simulink model "ofdm_txrx_mimo_2x"  (Simulink 6.6)
settings: Solver=VariableStepDiscrete  StartTime=0.0  StopTime=20e3  RelTol=1e-3
PreLoadFcn:
  | ofdm_rx_mimo_init
InitFcn:
  | ofdm_rx_mimo_init
StartFcn:
  | ofdm_rx_mimo_init
values from neighbouring files (symbols this model uses):
  BER_RAM_init_size = 2048    [ofdm_tx_mimo_init.m]
  CPLength = 16    [ofdm_tx_mimo_init.m]
  CRCPolynomial = hex2dec('04c11db7')    [ofdm_tx_mimo_init.m]
  freqOffset_track_filtCoef_i = hex2dec('2500')/2^32    [ofdm_rx_mimo_init.m]
  freqOffset_track_filtCoef_p = hex2dec('d0000')/2^32    [ofdm_rx_mimo_init.m]
  hi = zeros(1,length(cci))    [ofdm_rx_mimo_init.m]
  hr = zeros(1,length(ccr))    [ofdm_rx_mimo_init.m]
  max_CP_length = 16    [ofdm_tx_mimo_init.m]
  max_num_baseRateSymbols = 31    [ofdm_tx_mimo_init.m]
  max_num_subcarriers = 64    [ofdm_tx_mimo_init.m]
  max_num_trainingSymbols = 15    [ofdm_tx_mimo_init.m]
  max_numBytes = 16384    [ofdm_tx_mimo_init.m]
  max_OFDM_symbols = 2047    [ofdm_tx_mimo_init.m]
  modConstellation_qam16 = .75*[1 3 -1 -3]./3    [ofdm_tx_mimo_init.m]
  modConstellation_qam256 = 0.9375*[3 1 5 7 11 9 13 15 -3 -1 -5 -7 -11 -9 -13 -15]./15    [ofdm_tx_mimo_init.m]
  modConstellation_qam64 = 0.875*[3 1 5 7 -3 -1 -5 -7]./7    [ofdm_tx_mimo_init.m]
  modConstellation_qpsk = [1 -1]./sqrt(2)    [ofdm_tx_mimo_init.m]
  numBaseRateSymbols = 2    [ofdm_tx_mimo_init.m]
  numSubcarriers = 64    [ofdm_tx_mimo_init.m]
  numTrainingSymbols = 2    [ofdm_tx_mimo_init.m]
  pilot1_indicies = 7 + ( (64-7) * 2^16)    (= 3735559)    [ofdm_tx_mimo_init.m]
  pilot2_indicies = 21 + ( (64-21) * 2^16)    (= 2818069)    [ofdm_tx_mimo_init.m]
  pilotValue_neg = hex2dec('8000') + (2^16 * 0)    [ofdm_tx_mimo_init.m]
  pilotValue_pos = hex2dec('7FFF') + (2^16 * 0)    [ofdm_tx_mimo_init.m]
  pkt_crossCorr_thresh = 0.7    [ofdm_rx_mimo_init.m]
  pkt_energy_thresh = 0    [ofdm_rx_mimo_init.m]
  pkt_numPayloadBytes = 1400+42-6    (= 1436)    [ofdm_tx_mimo_init.m]
  pktByteNums = numHeaderBytes +  				byteIndex_numPayloadBytes(1)*2^8 +  				byteIndex_numPayloadBytes(2)*2^16 +  				byteIndex_simpleDynModMasks * 2^24    [ofdm_rx_mimo_init.m]
  PktDet_Delay = 26 + Symbol_Timing_Offset*2^7    (= 1050)    [ofdm_rx_mimo_init.m]
  preamble = 6*[0 shortsyms_10 longsyms_2]    [ofdm_tx_mimo_init.m]
  preamble_I = real(preamble)    [ofdm_tx_mimo_init.m]
  preamble_Q = imag(preamble)    [ofdm_tx_mimo_init.m]
  RAM_init_size = max_numBytes    (= 16384)    [ofdm_tx_mimo_init.m]
  reg_Interrupt_PktBuf_Control = reg_InterruptControl +  	1 * 2^16 +  	0 * 2^24    (= 65656)    [ofdm_rx_mimo_init.m]
  rx_controlBits = 1 * 2  		 + 1 * 4  		 + 0 * 8  		 + 1 * 16 * rx_SISO_Mode  		 + 1 * 32  		 + 1 * 64  		 + 0 * 128  		 + 1 * 256  		 + 0 * 512  		 + 1 * 1024  		 + 0 * 2048  		 + 1 * 4096  		 + 1 * 8192  		 + 0 * 16384  		 + 1 * 65536  		 + 1 * 131072  		 + 0 * 262144  		 + 1 * 524288    [ofdm_rx_mimo_init.m]
  Rx_PhaseNoiseTrack_K = 0.6094    [ofdm_rx_mimo_init.m]
  rx_postEq_scaling = round(rxScaling*2^11) * (1 + 2^16)    [ofdm_rx_mimo_init.m]
  RxDataScrambling_Seq = [40 198 78 63 82 173 102 245 48 111 172 115 147 230 216 93 72 65 62 2 205 242 122 90 128 83 105 97 73 10 5 252]    [ofdm_tx_mimo_init.m]
  simOnly_numSamples = length(preamble)+( (numSubcarriers+CPLength)*(numTrainingSymbols + numBaseRateSymbols + numFullRateSymbols/2) )    [ofdm_tx_mimo_init.m]
  train = [train train]    [ofdm_tx_mimo_init.m]
  tx_controlBits = (antB_preambleShift * 2^4) + (2^0 * tx_SISO_Mode) + 4*0 + 8*1    (= 73)    [ofdm_tx_mimo_init.m]
  TxDataScrambling_Seq = [40 198 78 63 82 173 102 245 48 111 172 115 147 230 216 93 72 65 62 2 205 242 122 90 128 83 105 97 73 10 5 252]    [ofdm_tx_mimo_init.m]
  txReg_symbolCounts = (2^16 * numFullRateSymbols) + (2^8 *numBaseRateSymbols ) + numTrainingSymbols    [ofdm_tx_mimo_init.m]
  TxRx_FFTScaling = bin2dec('010111') + (bin2dec('000101') * 2^6)    [ofdm_tx_mimo_init.m]
undefined symbols (not defined in the file or in its folder): AntA, AntB, BaseRate, CRC_table_gen, gcbh, intfilt, pktTiming_count_payloadStart, pktTiming_symbol_length, xlBlockLoadCallback, xlGetSimulinkPeriod
libraries: xbsIndex_r4 x3973, dspsigops x4, commchan3 x2, commrflib2 x2, dspsrcs4 x1
(part 4 of 38)

== system ofdm_txrx_mimo_2x/Memory-mapped Registers/Tx Registers ==
  note: LSB
  note: 0x1
  note: 0x2
  note: 0x2
  note: 0x4
  note: 0x8
  note: 0xF0
  note: 0x100
  note: 0x1
  Down Sample{1,3..5} (4 alike) : xbsIndex_r4/Down Sample  sample_ratio=2  sample_phase="Last Value of Frame  (most efficient)"  en=off  latency=1  dbl_ovrd=off  block_type=dsamp
      settings: block_version=VER_STRING_GOES_HERE
  Down Sample2 : xbsIndex_r4/Down Sample  sample_ratio=4  sample_phase="Last Value of Frame  (most efficient)"  en=off  latency=1  dbl_ovrd=off  block_type=dsamp
      settings: block_version=VER_STRING_GOES_HERE
  Read Only Register : Subsystem  AncestorBlock=|  mask{regName='Tx_PktRunning'}
      | WARP_Blockset/Register Wrappers
      | (Sysgen 10.1+)/Read Only Register
      mask prompts: regName "Register Name"
      mask help:
      | This block is a wrapper for System Generator's To Register shared memory block. This register is designed for use with WARP's sysgen2opb tool.
      |
      | Note: The Regisger Name paramter below must be unique for every instance of Read-Write and Read-Only registers in your system.
      mask init: same as ofdm_txrx_mimo_2x/Memory-mapped Registers/Rx Registers/Read Only Register
      settings: AttributesFormatString=RO Reg: %<regName>\n%<ReferenceBlock>
  Read-Write Register : Subsystem  AncestorBlock=|  mask{regName='Tx_Start_Reset_Control', out_dataType=Unsigned, out_numBits=32, out_binaryPt=0, initValue=0, sampPeriod=1}
      | WARP_Blockset/Register Wrappers
      | (Sysgen 10.1+)/Read-Write Register
      mask prompts: regName "Register Name"; out_dataType "Output Type"; out_numBits "Output Num Bits"; out_binaryPt "Output Binary Point"; initValue "Initial Value"; sampPeriod "Sample Period"
      mask help:
      | This block is a wrapper for two registers from the System Generator Shared Memory blockset:
      | a) A "From Register" block, which provides a write-only register for the host processor
      | b) A "To Register" block, which provides a read-only register for the host processor
      |
      | Note: The Regisger Name paramter below must be unique for every instance of shared-memory registers in your system. System Generator will throw an error if duplicate names are found.
      mask init: same as ofdm_txrx_mimo_2x/Memory-mapped Registers/Rx Registers/Read-Write Register
      settings: AttributesFormatString=RW Reg: %<regName>
      mask StyleString: edit,popup(Unsigned|Signed  (2's comp)),edit,edit,edit,edit
  Read-Write Register1 : Subsystem  AncestorBlock=|  mask{regName='Tx_ControlBits', out_dataType=Unsigned, out_numBits=32, out_binaryPt=0, initValue=tx_controlBits, sampPeriod=1}
      | WARP_Blockset/Register Wrappers
      | (Sysgen 10.1+)/Read-Write Register
      mask prompts: regName "Register Name"; out_dataType "Output Type"; out_numBits "Output Num Bits"; out_binaryPt "Output Binary Point"; initValue "Initial Value"; sampPeriod "Sample Period"
      mask help:
      | This block is a wrapper for two registers from the System Generator Shared Memory blockset:
      | a) A "From Register" block, which provides a write-only register for the host processor
      | b) A "To Register" block, which provides a read-only register for the host processor
      |
      | Note: The Regisger Name paramter below must be unique for every instance of shared-memory registers in your system. System Generator will throw an error if duplicate names are found.
      mask init: same as ofdm_txrx_mimo_2x/Memory-mapped Registers/Rx Registers/Read-Write Register
      settings: AttributesFormatString=RW Reg: %<regName>
      mask StyleString: edit,popup(Unsigned|Signed  (2's comp)),edit,edit,edit,edit
  Read-Write Register2 : Subsystem  AncestorBlock=|  mask{regName='Tx_OFDM_SymCounts', out_dataType=Unsigned, out_numBits=32, out_binaryPt=0, initValue=txReg_symbolCounts, sampPeriod=1}
      | WARP_Blockset/Register Wrappers
      | (Sysgen 10.1+)/Read-Write Register
      mask prompts: regName "Register Name"; out_dataType "Output Type"; out_numBits "Output Num Bits"; out_binaryPt "Output Binary Point"; initValue "Initial Value"; sampPeriod "Sample Period"
      mask help:
      | This block is a wrapper for two registers from the System Generator Shared Memory blockset:
      | a) A "From Register" block, which provides a write-only register for the host processor
      | b) A "To Register" block, which provides a read-only register for the host processor
      |
      | Note: The Regisger Name paramter below must be unique for every instance of shared-memory registers in your system. System Generator will throw an error if duplicate names are found.
      mask init: same as ofdm_txrx_mimo_2x/Memory-mapped Registers/Rx Registers/Read-Write Register
      settings: AttributesFormatString=RW Reg: %<regName>
      mask StyleString: edit,popup(Unsigned|Signed  (2's comp)),edit,edit,edit,edit
  Reinterpret : xbsIndex_r4/Reinterpret  force_arith_type=on  arith_type=Unsigned  force_bin_pt=on  bin_pt=16  block_type=reinterpret
      settings: infoedit="Changes signal type without altering the binary representation.   You can changed the signal between signed and unsigned, and relocate the binary point.<P><P>Hardware notes: In hardware this block costs nothing.<P><P>Example:  Suppose the input is 6 bits wide, signed, with 2 fractional bits, and the output is forced to unsigned with 0 fractional bits.  Then an input of -2.0 (1110.00 in binary 2's complement) becomes an output of 56 (111000 in binary)."  block_version=10.1
  Slice1 : xbsIndex_r4/Slice  nbits=1  boolean_output=on  mode=Lower Bit Location + Width  bit1=0  base1=MSB of Input  bit0=0  base0=LSB of Input  dbl_ovrd=off  block_type=slice
      settings: infoedit="Extracts a given range of bits from each input sample and presents it at the output.  The output type is ordinarily unsigned with binary point at zero, but can be Boolean when the slice is one bit wide.<P><P>Hardware notes: In hardware this block costs nothing."  block_version=VER_STRING_GOES_HERE
  Slice14 : xbsIndex_r4/Slice  nbits=1  boolean_output=off  mode=Lower Bit Location + Width  bit1=0  base1=MSB of Input  bit0=0  base0=LSB of Input  dbl_ovrd=off  block_type=slice
      settings: infoedit="Extracts a given range of bits from each input sample and presents it at the output.  The output type is ordinarily unsigned with binary point at zero, but can be Boolean when the slice is one bit wide.<P><P>Hardware notes: In hardware this block costs nothing."  block_version=VER_STRING_GOES_HERE
  Slice15 : xbsIndex_r4/Slice  nbits=1  boolean_output=on  mode=Lower Bit Location + Width  bit1=0  base1=MSB of Input  bit0=1  base0=LSB of Input  dbl_ovrd=off  block_type=slice
      settings: infoedit="Extracts a given range of bits from each input sample and presents it at the output.  The output type is ordinarily unsigned with binary point at zero, but can be Boolean when the slice is one bit wide.<P><P>Hardware notes: In hardware this block costs nothing."  block_version=VER_STRING_GOES_HERE
  Slice3 : xbsIndex_r4/Slice  nbits=1  boolean_output=on  mode=Lower Bit Location + Width  bit1=0  base1=MSB of Input  bit0=2  base0=LSB of Input  dbl_ovrd=off  block_type=slice
      settings: infoedit="Extracts a given range of bits from each input sample and presents it at the output.  The output type is ordinarily unsigned with binary point at zero, but can be Boolean when the slice is one bit wide.<P><P>Hardware notes: In hardware this block costs nothing."  block_version=VER_STRING_GOES_HERE
  Slice4 : xbsIndex_r4/Slice  nbits=1  boolean_output=on  mode=Lower Bit Location + Width  bit1=0  base1=MSB of Input  bit0=3  base0=LSB of Input  dbl_ovrd=off  block_type=slice
      settings: infoedit="Extracts a given range of bits from each input sample and presents it at the output.  The output type is ordinarily unsigned with binary point at zero, but can be Boolean when the slice is one bit wide.<P><P>Hardware notes: In hardware this block costs nothing."  block_version=VER_STRING_GOES_HERE
  Slice5 : xbsIndex_r4/Slice  nbits=4  boolean_output=off  mode=Lower Bit Location + Width  bit1=0  base1=MSB of Input  bit0=4  base0=LSB of Input  dbl_ovrd=off  block_type=slice
      settings: infoedit="Extracts a given range of bits from each input sample and presents it at the output.  The output type is ordinarily unsigned with binary point at zero, but can be Boolean when the slice is one bit wide.<P><P>Hardware notes: In hardware this block costs nothing."  block_version=VER_STRING_GOES_HERE
  Slice6 : xbsIndex_r4/Slice  nbits=1  boolean_output=off  mode=Lower Bit Location + Width  bit1=0  base1=MSB of Input  bit0=8  base0=LSB of Input  dbl_ovrd=off  block_type=slice
      settings: infoedit="Extracts a given range of bits from each input sample and presents it at the output.  The output type is ordinarily unsigned with binary point at zero, but can be Boolean when the slice is one bit wide.<P><P>Hardware notes: In hardware this block costs nothing."  block_version=VER_STRING_GOES_HERE
  Write-Only Register : Subsystem  AncestorBlock=|  mask{regName='Tx_PreambleMasking', out_dataType=Unsigned, out_numBits=32, out_binaryPt=0, initValue=0, sampPeriod=1}
      | WARP_Blockset/Register Wrappers
      | (Sysgen 10.1+)/Write-Only Register
      mask prompts: regName "Register Name"; out_dataType "Output Type"; out_numBits "Output Num Bits"; out_binaryPt "Output Binary Point"; initValue "Initial Value"; sampPeriod "Sample Period"
      mask help:
      | This block is a wrapper for System Generator's "From Register" shared memory block.
      |
      | Note: The Regisger Name paramter below must be unique for every instance of shared-memory registers in your system. System Generator will throw an error if duplicate names are found.
      mask init: same as ofdm_txrx_mimo_2x/Memory-mapped Registers/Rx Registers/Write-Only Register
      settings: AttributesFormatString=RW Reg: %<regName>
      mask StyleString: edit,popup(Unsigned|Signed  (2's comp)),edit,edit,edit,edit
  Write-Only Register1 : Subsystem  AncestorBlock=|  mask{regName='Tx_PreambleScaling', out_dataType=Unsigned, out_numBits=16, out_binaryPt=0, initValue=0.2539 * 2^16, sampPeriod=1}
      | WARP_Blockset/Register Wrappers
      | (Sysgen 10.1+)/Write-Only Register
      mask prompts: regName "Register Name"; out_dataType "Output Type"; out_numBits "Output Num Bits"; out_binaryPt "Output Binary Point"; initValue "Initial Value"; sampPeriod "Sample Period"
      mask help:
      | This block is a wrapper for System Generator's "From Register" shared memory block.
      |
      | Note: The Regisger Name paramter below must be unique for every instance of shared-memory registers in your system. System Generator will throw an error if duplicate names are found.
      mask init: same as ofdm_txrx_mimo_2x/Memory-mapped Registers/Rx Registers/Write-Only Register
      settings: AttributesFormatString=RW Reg: %<regName>
      mask StyleString: edit,popup(Unsigned|Signed  (2's comp)),edit,edit,edit,edit
  Write-Only Register2 : Subsystem  AncestorBlock=|  mask{regName='Tx_Pilots_Index1', out_dataType=Unsigned, out_numBits=32, out_binaryPt=0, initValue=pilot1_indicies, sampPeriod=1}
      | WARP_Blockset/Register Wrappers
      | (Sysgen 10.1+)/Write-Only Register
      mask prompts: regName "Register Name"; out_dataType "Output Type"; out_numBits "Output Num Bits"; out_binaryPt "Output Binary Point"; initValue "Initial Value"; sampPeriod "Sample Period"
      mask help:
      | This block is a wrapper for System Generator's "From Register" shared memory block.
      |
      | Note: The Regisger Name paramter below must be unique for every instance of shared-memory registers in your system. System Generator will throw an error if duplicate names are found.
      mask init: same as ofdm_txrx_mimo_2x/Memory-mapped Registers/Rx Registers/Write-Only Register
      settings: AttributesFormatString=RW Reg: %<regName>
      mask StyleString: edit,popup(Unsigned|Signed  (2's comp)),edit,edit,edit,edit
  Write-Only Register3 : Subsystem  AncestorBlock=|  mask{regName='Tx_Pilots_Index2', out_dataType=Unsigned, out_numBits=32, out_binaryPt=0, initValue=pilot2_indicies, sampPeriod=1}
      | WARP_Blockset/Register Wrappers
      | (Sysgen 10.1+)/Write-Only Register
      mask prompts: regName "Register Name"; out_dataType "Output Type"; out_numBits "Output Num Bits"; out_binaryPt "Output Binary Point"; initValue "Initial Value"; sampPeriod "Sample Period"
      mask help:
      | This block is a wrapper for System Generator's "From Register" shared memory block.
      |
      | Note: The Regisger Name paramter below must be unique for every instance of shared-memory registers in your system. System Generator will throw an error if duplicate names are found.
      mask init: same as ofdm_txrx_mimo_2x/Memory-mapped Registers/Rx Registers/Write-Only Register
      settings: AttributesFormatString=RW Reg: %<regName>
      mask StyleString: edit,popup(Unsigned|Signed  (2's comp)),edit,edit,edit,edit
  Write-Only Register4 : Subsystem  AncestorBlock=|  mask{regName='Tx_Pilots_Value1', out_dataType=Unsigned, out_numBits=32, out_binaryPt=0, initValue=pilotValue_neg, sampPeriod=1}
      | WARP_Blockset/Register Wrappers
      | (Sysgen 10.1+)/Write-Only Register
      mask prompts: regName "Register Name"; out_dataType "Output Type"; out_numBits "Output Num Bits"; out_binaryPt "Output Binary Point"; initValue "Initial Value"; sampPeriod "Sample Period"
      mask help:
      | This block is a wrapper for System Generator's "From Register" shared memory block.
      |
      | Note: The Regisger Name paramter below must be unique for every instance of shared-memory registers in your system. System Generator will throw an error if duplicate names are found.
      mask init: same as ofdm_txrx_mimo_2x/Memory-mapped Registers/Rx Registers/Write-Only Register
      settings: AttributesFormatString=RW Reg: %<regName>
      mask StyleString: edit,popup(Unsigned|Signed  (2's comp)),edit,edit,edit,edit
  Write-Only Register5 : Subsystem  AncestorBlock=|  mask{regName='Tx_Pilots_Value2', out_dataType=Unsigned, out_numBits=32, out_binaryPt=0, initValue=pilotValue_pos, sampPeriod=1}
      | WARP_Blockset/Register Wrappers
      | (Sysgen 10.1+)/Write-Only Register
      mask prompts: regName "Register Name"; out_dataType "Output Type"; out_numBits "Output Num Bits"; out_binaryPt "Output Binary Point"; initValue "Initial Value"; sampPeriod "Sample Period"
      mask help:
      | This block is a wrapper for System Generator's "From Register" shared memory block.
      |
      | Note: The Regisger Name paramter below must be unique for every instance of shared-memory registers in your system. System Generator will throw an error if duplicate names are found.
      mask init: same as ofdm_txrx_mimo_2x/Memory-mapped Registers/Rx Registers/Write-Only Register
      settings: AttributesFormatString=RW Reg: %<regName>
      mask StyleString: edit,popup(Unsigned|Signed  (2's comp)),edit,edit,edit,edit
  tag scope: TxReg_AntBPreambleShift=global, TxReg_DisableAntBPreamble=global, TxReg_OFDM_SymCounts=global, TxReg_PilotScrambling=global, TxReg_PreambleMasking=global, TxReg_PreambleScaling=global, TxReg_SISO_AntB=global, TxReg_SISO_Mode=global, TxReg_TxReset=global, TxReg_TxStart=global, regTxRx_PilotToneIndex1=global, regTxRx_PilotToneIndex2=global, regTx_PilotToneValue1=global, regTx_PilotToneValue2=global
  wires:
  Read-Write Register1/1(D) -> Down Sample1/1
  Slice1/1 -> [TxReg_SISO_Mode]
  Slice14/1 -> [TxReg_TxReset]
  Read-Write Register/1(D) -> Down Sample3/1
  Slice15/1 -> [TxReg_TxStart]
  Down Sample3/1 -> Slice14/1, Slice15/1
  Down Sample1/1 -> Slice1/1, Slice3/1, Slice4/1, Slice5/1, Slice6/1
  Slice3/1 -> [TxReg_DisableAntBPreamble]
  Slice4/1 -> [TxReg_PilotScrambling]
  Slice5/1 -> [TxReg_AntBPreambleShift]
  Slice6/1 -> [TxReg_SISO_AntB]
  Write-Only Register/1(D) -> Down Sample2/1
  Down Sample2/1 -> [TxReg_PreambleMasking]
  Read-Write Register2/1(D) -> [TxReg_OFDM_SymCounts]
  Write-Only Register1/1(D) -> Reinterpret/1
  Write-Only Register2/1(D) -> Down Sample4/1
  Write-Only Register3/1(D) -> Down Sample5/1
  Down Sample4/1 -> [regTxRx_PilotToneIndex1]
  Down Sample5/1 -> [regTxRx_PilotToneIndex2]
  Write-Only Register4/1(D) -> [regTx_PilotToneValue1]
  Write-Only Register5/1(D) -> [regTx_PilotToneValue2]
  [regTx_PktRunning] -> Read Only Register/1(D)
  [regTx_PktRunningEn] -> Read Only Register/2(En)
  Reinterpret/1 -> [TxReg_PreambleScaling]

== system ofdm_txrx_mimo_2x/Memory-mapped Registers/TxRx Registers ==
  16LSB+8 : xbsIndex_r4/Slice  nbits=16  boolean_output=off  mode=Lower Bit Location + Width  bit1=0  base1=MSB of Input  bit0=8  base0=LSB of Input  dbl_ovrd=off  block_type=slice
      settings: infoedit="Extracts a given range of bits from each input sample and presents it at the output.  The output type is ordinarily unsigned with binary point at zero, but can be Boolean when the slice is one bit wide.<P><P>Hardware notes: In hardware this block costs nothing."  block_version=VER_STRING_GOES_HERE
  6LSB+6 : xbsIndex_r4/Slice  nbits=6  boolean_output=off  mode=Lower Bit Location + Width  bit1=0  base1=MSB of Input  bit0=6  base0=LSB of Input  dbl_ovrd=off  block_type=slice
      settings: infoedit="Extracts a given range of bits from each input sample and presents it at the output.  The output type is ordinarily unsigned with binary point at zero, but can be Boolean when the slice is one bit wide.<P><P>Hardware notes: In hardware this block costs nothing."  block_version=VER_STRING_GOES_HERE
  6LSB1 : xbsIndex_r4/Slice  nbits=6  boolean_output=off  mode=Lower Bit Location + Width  bit1=0  base1=MSB of Input  bit0=0  base0=LSB of Input  dbl_ovrd=off  block_type=slice
      settings: infoedit="Extracts a given range of bits from each input sample and presents it at the output.  The output type is ordinarily unsigned with binary point at zero, but can be Boolean when the slice is one bit wide.<P><P>Hardware notes: In hardware this block costs nothing."  block_version=VER_STRING_GOES_HERE
  8LSB : xbsIndex_r4/Slice  nbits=8  boolean_output=off  mode=Lower Bit Location + Width  bit1=0  base1=MSB of Input  bit0=0  base0=LSB of Input  dbl_ovrd=off  block_type=slice
      settings: infoedit="Extracts a given range of bits from each input sample and presents it at the output.  The output type is ordinarily unsigned with binary point at zero, but can be Boolean when the slice is one bit wide.<P><P>Hardware notes: In hardware this block costs nothing."  block_version=VER_STRING_GOES_HERE
  Down Sample25 : xbsIndex_r4/Down Sample  sample_ratio=2  sample_phase="Last Value of Frame  (most efficient)"  en=off  latency=1  dbl_ovrd=off  block_type=dsamp
      settings: block_version=VER_STRING_GOES_HERE
  Down Sample7 : xbsIndex_r4/Down Sample  sample_ratio=2  sample_phase="Last Value of Frame  (most efficient)"  en=off  latency=1  dbl_ovrd=off  block_type=dsamp
      settings: block_version=VER_STRING_GOES_HERE
  InterruptControl : Subsystem
  Read-Write Register : Subsystem  AncestorBlock=|  mask{regName='TxRx_Interrupt_PktBuf_Ctrl', out_dataType=Unsigned, out_numBits=32, out_binaryPt=0, initValue=reg_Interrupt_PktBuf_Control, sampPeriod=1}
      | WARP_Blockset/Register Wrappers
      | (Sysgen 10.1+)/Read-Write Register
      mask prompts: regName "Register Name"; out_dataType "Output Type"; out_numBits "Output Num Bits"; out_binaryPt "Output Binary Point"; initValue "Initial Value"; sampPeriod "Sample Period"
      mask help:
      | This block is a wrapper for two registers from the System Generator Shared Memory blockset:
      | a) A "From Register" block, which provides a write-only register for the host processor
      | b) A "To Register" block, which provides a read-only register for the host processor
      |
      | Note: The Regisger Name paramter below must be unique for every instance of shared-memory registers in your system. System Generator will throw an error if duplicate names are found.
      mask init: same as ofdm_txrx_mimo_2x/Memory-mapped Registers/Rx Registers/Read-Write Register
      settings: AttributesFormatString=RW Reg: %<regName>
      mask StyleString: edit,popup(Unsigned|Signed  (2's comp)),edit,edit,edit,edit
  Write-Only Register : Subsystem  AncestorBlock=|  mask{regName='TxRx_FFT_Scaling', out_dataType=Unsigned, out_numBits=12, out_binaryPt=0, initValue=TxRx_FFTScaling, sampPeriod=1}
      | WARP_Blockset/Register Wrappers
      | (Sysgen 10.1+)/Write-Only Register
      mask prompts: regName "Register Name"; out_dataType "Output Type"; out_numBits "Output Num Bits"; out_binaryPt "Output Binary Point"; initValue "Initial Value"; sampPeriod "Sample Period"
      mask help:
      | This block is a wrapper for System Generator's "From Register" shared memory block.
      |
      | Note: The Regisger Name paramter below must be unique for every instance of shared-memory registers in your system. System Generator will throw an error if duplicate names are found.
      mask init: same as ofdm_txrx_mimo_2x/Memory-mapped Registers/Rx Registers/Write-Only Register
      settings: AttributesFormatString=RW Reg: %<regName>
      mask StyleString: edit,popup(Unsigned|Signed  (2's comp)),edit,edit,edit,edit
  tag scope: Rx_FFTScaling=global, Tx_FFTScaling=global, regTxRx_PktBuffOffsets=global
  wires:
  Read-Write Register/1(D) -> Down Sample7/1, 16LSB+8/1
  Down Sample7/1 -> 8LSB/1
  Write-Only Register/1(D) -> Down Sample25/1
  Down Sample25/1 -> 6LSB1/1, 6LSB+6/1
  6LSB1/1 -> [Tx_FFTScaling]
  6LSB+6/1 -> [Rx_FFTScaling]
  16LSB+8/1 -> [regTxRx_PktBuffOffsets]
  8LSB/1 -> InterruptControl/1(Reg_8b)

== system ofdm_txrx_mimo_2x/OFDM Rx MIMO/ADC Inputs & Antenna Selection ==
  "A I " : Inport
  "A Q " : Inport  Port=2
  "B I " : Inport  Port=3
  "B Q " : Inport  Port=4
  PktDetReset : Inport  Port=5
  Antenna Selection : Subsystem
  Down Sample{,1..3} (4 alike) : xbsIndex_r4/Down Sample  sample_ratio=2  sample_phase="Last Value of Frame  (most efficient)"  en=off  latency=1  dbl_ovrd=off  block_type=dsamp
      settings: block_version=8.2.02
  Rx_AntA_ADCI : xbsIndex_r4/Gateway In  arith_type="Signed  (2's comp)"  n_bits=14  bin_pt=13  quantization="Round  (unbiased: +/- Inf)"  overflow=Saturate  period=2  dbl_ovrd=off  timing_constraint=None  locs_specified=off  LOCs={}  block_type=gatewayin
      settings: infoedit="Gateway in block.  Converts inputs of type Simulink integer, double and fixed point to  Xilinx fixed point type.<P><P>Hardware notes:  In hardware these blocks become top level input ports."  block_version=VER_STRING_GOES_HERE
  Rx_AntA_ADCQ : xbsIndex_r4/Gateway In  arith_type="Signed  (2's comp)"  n_bits=14  bin_pt=13  quantization="Round  (unbiased: +/- Inf)"  overflow=Saturate  period=2  dbl_ovrd=off  timing_constraint=None  locs_specified=off  LOCs={}  block_type=gatewayin
      settings: infoedit="Gateway in block.  Converts inputs of type Simulink integer, double and fixed point to  Xilinx fixed point type.<P><P>Hardware notes:  In hardware these blocks become top level input ports."  block_version=VER_STRING_GOES_HERE
  Rx_AntB_ADCI : xbsIndex_r4/Gateway In  arith_type="Signed  (2's comp)"  n_bits=14  bin_pt=13  quantization="Round  (unbiased: +/- Inf)"  overflow=Saturate  period=2  dbl_ovrd=off  timing_constraint=None  locs_specified=off  LOCs={}  block_type=gatewayin
      settings: infoedit="Gateway in block.  Converts inputs of type Simulink integer, double and fixed point to  Xilinx fixed point type.<P><P>Hardware notes:  In hardware these blocks become top level input ports."  block_version=VER_STRING_GOES_HERE
  Rx_AntB_ADCQ : xbsIndex_r4/Gateway In  arith_type="Signed  (2's comp)"  n_bits=14  bin_pt=13  quantization="Round  (unbiased: +/- Inf)"  overflow=Saturate  period=2  dbl_ovrd=off  timing_constraint=None  locs_specified=off  LOCs={}  block_type=gatewayin
      settings: infoedit="Gateway in block.  Converts inputs of type Simulink integer, double and fixed point to  Xilinx fixed point type.<P><P>Hardware notes:  In hardware these blocks become top level input ports."  block_version=VER_STRING_GOES_HERE
  A I : Outport
  A Q : Outport  Port=2
  B I : Outport  Port=3
  B Q : Outport  Port=4
  tag scope: AntA_ADC_I_Filt=global, AntA_ADC_Q_Filt=global
  wires:
  "B Q "/1 -> Rx_AntB_ADCQ/1
  "B I "/1 -> Rx_AntB_ADCI/1
  "A Q "/1 -> Rx_AntA_ADCQ/1
  "A I "/1 -> Rx_AntA_ADCI/1
  Rx_AntA_ADCI/1 -> Down Sample/1
  Rx_AntB_ADCQ/1 -> Down Sample3/1
  Rx_AntB_ADCI/1 -> Down Sample2/1
  Rx_AntA_ADCQ/1 -> Down Sample1/1
  Down Sample/1 -> Antenna Selection/1(AntA_I)
  Down Sample1/1 -> Antenna Selection/2(AntA_Q)
  Down Sample2/1 -> Antenna Selection/3(AntB_I)
  Down Sample3/1 -> Antenna Selection/4(AntB_Q)
  Antenna Selection/1(A I) -> A I/1, [AntA_ADC_I_Filt]
  Antenna Selection/2(A Q) -> A Q/1, [AntA_ADC_Q_Filt]
  Antenna Selection/3(B I) -> B I/1
  Antenna Selection/4(B Q) -> B Q/1
  PktDetReset/1 -> Antenna Selection/5(PktDetReset)

== system ofdm_txrx_mimo_2x/OFDM Rx MIMO/Coarse Freq Correction ==
  Reset : Inport
  PktDet : Inport  Port=2
  AntA I : Inport  Port=3
  AntA Q : Inport  Port=4
  AntB I : Inport  Port=5
  AntB Q : Inport  Port=6
  Fine Freq : Inport  Port=7
  A*B : Subsystem
  A*B1 : Subsystem
  Add1 : xbsIndex_r4/AddSub  mode=Addition  use_carryin=off  use_carryout=off  en=off  latency=0  precision=User Defined  arith_type=Unsigned  n_bits=32  bin_pt=32  quantization=Truncate  overflow=Wrap  dbl_ovrd=off  use_behavioral_HDL=on  pipelined=off  use_rpm=on  hw_selection=Fabric  block_type=addsub
      settings: xl_area=[9 0 0 16 0 0 0]  block_version=VER_STRING_GOES_HERE
  Bus Creator1 : BusCreator  Inputs=2
  Coarse Freq Offset Calc Combo : Subsystem
  Complex to Magnitude-Angle : ComplexToMagnitudeAngle
  Complex to Real-Imag : ComplexToRealImag
  Convert{,1..3} (4 alike) : xbsIndex_r4/Convert  arith_type="Signed  (2's comp)"  n_bits=14  bin_pt=13  quantization=Truncate  overflow=Flag as error  latency=0  dbl_ovrd=off  pipeline=off  block_type=convert
      settings: xl_area=[0 0 0 0 0 0 0]  block_version=VER_STRING_GOES_HERE
  DDS : Subsystem
  Down Sample : xbsIndex_r4/Down Sample  sample_ratio=2  sample_phase="Last Value of Frame  (most efficient)"  en=off  latency=1  dbl_ovrd=off  block_type=dsamp
      settings: block_version=VER_STRING_GOES_HERE
  Freq Correction Sinusoids : Scope  NumInputPorts=5
      settings: TimeRange=5000  YMin=0~-1e-006~-1~5.55112e-017~0.2  YMax=1~3e-007~1~1~1.5  SaveName=ScopeData11
  Gateway Out{,1..3} (4 alike) : xbsIndex_r4/Gateway Out  hdl_port=off  timing_constraint=None  locs_specified=off  LOCs={}  block_type=gatewayout
      settings: infoedit="Gateway out block.  Converts Xilinx fixed point inputs into ouputs of type Simulink integer, double, or fixed point.<P><P>Hardware notes:  In hardware these blocks become top level output ports or are discarded, depending on how they are configured."  xl_area=[0 0 0 0 0 0 0]  block_version=VER_STRING_GOES_HERE
  Mux2 : Mux  Inputs=2
  Product : Product  RndMeth=Zero
  Real-Imag to Complex : RealImagToComplex
  inv 2pi1 : xbsIndex_r4/Constant  arith_type=Unsigned  const=1-( (1-0.9996650654855521)/2)  n_bits=32  bin_pt=32  explicit_period=off  period=1  equ=P=C  opselect=C  inp2=PCIN>>17  opr=+  inp1=P  carry=CIN  dbl_ovrd=off  block_type=constant
      settings: block_version=VER_STRING_GOES_HERE
  "AntA I " : Outport
  "AntA Q " : Outport  Port=2
  "AntB I " : Outport  Port=3
  "AntB Q " : Outport  Port=4
  tag scope: CFO_PhaseInc=global
  wires:
  A*B/1(Re) -> Convert/1
  A*B/2(Im1) -> Convert1/1
  A*B1/1(Re) -> Convert2/1
  A*B1/2(Im1) -> Convert3/1
  DDS/1(Q) -> Gateway Out/1, A*B1/2(Im[B]), A*B/3(Im[B])
  DDS/2(I) -> Gateway Out1/1, A*B1/1(Re[B]), A*B/4(Re[B])
  Gateway Out1/1 -> Mux2/1, Real-Imag to Complex/1
  Gateway Out/1 -> Mux2/2, Real-Imag to Complex/2
  Mux2/1 -> Freq Correction Sinusoids/3
  Bus Creator1/1 -> Freq Correction Sinusoids/4
  [freqOffset] -> Complex to Real-Imag/1, Product/2
  Complex to Real-Imag/1 -> Bus Creator1/1
  Complex to Real-Imag/2 -> Bus Creator1/2
  Gateway Out2/1 -> Freq Correction Sinusoids/2
  Gateway Out3/1 -> Freq Correction Sinusoids/1
  AntB I/1 -> A*B1/3(Re[A])
  AntB Q/1 -> A*B1/4(Im[A])
  AntA I/1 -> A*B/1(Re[A])
  AntA Q/1 -> A*B/2(Im[A])
  Convert/1 -> "AntA I "/1, Coarse Freq Offset Calc Combo/1(I In)
  Convert1/1 -> "AntA Q "/1, Coarse Freq Offset Calc Combo/2(Q In)
  Real-Imag to Complex/1 -> Product/1
  Product/1 -> Complex to Magnitude-Angle/1
  Complex to Magnitude-Angle/2 -> Freq Correction Sinusoids/5
  Down Sample/1 -> Gateway Out2/1, Add1/2
  Fine Freq/1 -> Down Sample/1
  Add1/1 -> [CFO_PhaseInc], Gateway Out3/1, DDS/1(PhaseInc)
  Convert2/1 -> "AntB I "/1
  Convert3/1 -> "AntB Q "/1
  Reset/1 -> DDS/2(Rst), Coarse Freq Offset Calc Combo/3(Reset)
  PktDet/1 -> Coarse Freq Offset Calc Combo/4(PktDet)
  Coarse Freq Offset Calc Combo/1(Phase Inc) -> Add1/1

== system ofdm_txrx_mimo_2x/OFDM Rx MIMO/Equalizer & Packetizer ==
  Payload : Inport
  Y_I : Inport  Port=2
  Y_Q : Inport  Port=3
  H-I : Inport  Port=4
  H-Q : Inport  Port=5
  SubCar Index : Inport  Port=6
  Sym Index : Inport  Port=7
  Vin : Inport  Port=8
  CFO Loop Filter : Subsystem
  Convert{1,3..5} (4 alike) : xbsIndex_r4/Convert  arith_type="Signed  (2's comp)"  n_bits=14  bin_pt=13  quantization=Truncate  overflow=Wrap  latency=0  dbl_ovrd=off  pipeline=off  block_type=convert
      settings: xl_area=[0, 0, 0, 0, 0, 0, 0]  block_version=VER_STRING_GOES_HERE
  Equalizer : Subsystem
  Packet_Constructor : Subsystem
  Phase Adjust : Subsystem
  Phase Noise Tracker : Subsystem
  Pilot_Extractor : Subsystem
  Pkt Constructor Inputs : Scope  NumInputPorts=6
      settings: TimeRange=1800  YMin=-1~-0.8~0~0~19~-1  YMax=0.75~0.8~65~1~20~1  SaveName=ScopeData29
  Rx_debug_EQ_I : xbsIndex_r4/Gateway Out  hdl_port=on  timing_constraint=None  locs_specified=off  LOCs={}  block_type=gatewayout
      settings: infoedit="Gateway out block.  Converts Xilinx fixed point inputs into ouputs of type Simulink integer, double, or fixed point.<P><P>Hardware notes:  In hardware these blocks become top level output ports or are discarded, depending on how they are configured."  xl_area=[0 0 0 0 1 0 0]  block_version=VER_STRING_GOES_HERE
  Rx_debug_EQ_Q : xbsIndex_r4/Gateway Out  hdl_port=on  timing_constraint=None  locs_specified=off  LOCs={}  block_type=gatewayout
      settings: infoedit="Gateway out block.  Converts Xilinx fixed point inputs into ouputs of type Simulink integer, double, or fixed point.<P><P>Hardware notes:  In hardware these blocks become top level output ports or are discarded, depending on how they are configured."  xl_area=[0 0 0 0 1 0 0]  block_version=VER_STRING_GOES_HERE
  Rx_debug_PhaseCorrect : xbsIndex_r4/Gateway Out  hdl_port=on  timing_constraint=None  locs_specified=off  LOCs={}  block_type=gatewayout
      settings: infoedit="Gateway out block.  Converts Xilinx fixed point inputs into ouputs of type Simulink integer, double, or fixed point.<P><P>Hardware notes:  In hardware these blocks become top level output ports or are discarded, depending on how they are configured."  xl_area=[0 0 0 0 1 0 0]  block_version=VER_STRING_GOES_HERE
  Rx_debug_PhaseError : xbsIndex_r4/Gateway Out  hdl_port=on  timing_constraint=None  locs_specified=off  LOCs={}  block_type=gatewayout
      settings: infoedit="Gateway out block.  Converts Xilinx fixed point inputs into ouputs of type Simulink integer, double, or fixed point.<P><P>Hardware notes:  In hardware these blocks become top level output ports or are discarded, depending on how they are configured."  xl_area=[0 0 0 0 1 0 0]  block_version=VER_STRING_GOES_HERE
  Scaling : Subsystem
  Up Sample : xbsIndex_r4/Up Sample  sample_ratio=2  copy_samples=on  dbl_ovrd=off  block_type=usamp
      settings: infoedit="Up samples input data.  Inserted values  can be zeros or copies of the most recent input sample.<P><P>Hardware notes: No hardware is needed if inserted values are copies of the input sample; otherwise, a mux and single bit flip-flop are used."  block_version=8.2.02
  Up Sample1 : xbsIndex_r4/Up Sample  sample_ratio=2  copy_samples=on  dbl_ovrd=off  block_type=usamp
      settings: infoedit="Up samples input data.  Inserted values  can be zeros or copies of the most recent input sample.<P><P>Hardware notes: No hardware is needed if inserted values are copies of the input sample; otherwise, a mux and single bit flip-flop are used."  block_version=8.2.02
  Up Sample2 : xbsIndex_r4/Up Sample  sample_ratio=2  copy_samples=on  dbl_ovrd=off  block_type=usamp
      settings: infoedit="Up samples input data.  Inserted values  can be zeros or copies of the most recent input sample.<P><P>Hardware notes: No hardware is needed if inserted values are copies of the input sample; otherwise, a mux and single bit flip-flop are used."  block_version=8.2.02
  done{1,6..10} (6 alike) : xbsIndex_r4/Gateway Out  hdl_port=off  timing_constraint=None  locs_specified=off  LOCs={}  block_type=gatewayout
      settings: infoedit="Gateway out block.  Converts Xilinx fixed point inputs into ouputs of type Simulink integer, double, or fixed point.<P><P>Hardware notes:  In hardware these blocks become top level output ports or are discarded, depending on how they are configured."  xl_area=[0 0 0 0 1 0 0]  block_version=VER_STRING_GOES_HERE
  posedge : Subsystem
  Fine Freq : Outport
  done : Outport  Port=2
  tag scope: PilotPhaseErr=global
  wires:
  SubCar Index/1 -> Equalizer/7(SubCar Index)
  Sym Index/1 -> Equalizer/6(Sym Index)
  Scaling/1(I) -> Packet_Constructor/1(I), done6/1, Convert4/1
  Scaling/2(Q) -> done7/1, Packet_Constructor/2(Q), Convert5/1
  Scaling/3(" Xk_index") -> Packet_Constructor/3(Xk_index), done1/1
  Scaling/4(Vout) -> done8/1, Packet_Constructor/4(Vin)
  Scaling/5(" Sym Index") -> Packet_Constructor/5(Sym Index), done9/1
  Packet_Constructor/1(done) -> done/1, Pilot_Extractor/1(rst)
  done6/1 -> Pkt Constructor Inputs/1
  done7/1 -> Pkt Constructor Inputs/2
  done1/1 -> Pkt Constructor Inputs/3
  done8/1 -> Pkt Constructor Inputs/4
  done9/1 -> Pkt Constructor Inputs/5
  H-I/1 -> Equalizer/3(H-I)
  H-Q/1 -> Equalizer/4(H-Q)
  Vin/1 -> Equalizer/5(Vin)
  Y_I/1 -> Equalizer/1(Y_I)
  Y_Q/1 -> Equalizer/2(Y_Q)
  Payload/1 -> posedge/1(D)
  posedge/1(Q) -> Packet_Constructor/6(Start), done10/1
  done10/1 -> Pkt Constructor Inputs/6
  Equalizer/1(I-eq) -> Phase Adjust/2(I), Pilot_Extractor/2(Eq-I)
  Equalizer/2(Q-eq) -> Phase Adjust/3(Q), Pilot_Extractor/3(Eq-Q)
  Equalizer/3(" Xk_index") -> Phase Adjust/4(SubCar Index), Pilot_Extractor/4(Xk_index)
  Equalizer/4(Vout) -> Phase Adjust/5(Vin)
  Equalizer/5(" Sym Index") -> Phase Adjust/6(Sym Index), Pilot_Extractor/5(Sym Index), Phase Noise Tracker/4(Sym Index)
  Pilot_Extractor/1(Phase Err) -> Phase Noise Tracker/1(Phase Err), CFO Loop Filter/1(Phase Err), [PilotPhaseErr], Convert3/1
  Pilot_Extractor/2(Rst) -> Phase Noise Tracker/2(Reset), CFO Loop Filter/2(Rst)
  Pilot_Extractor/3(Vout) -> Phase Noise Tracker/3(Vin), CFO Loop Filter/3(Vin)
  Phase Noise Tracker/1(theta) -> Phase Adjust/1(Phase), Convert1/1
  CFO Loop Filter/1(Fine Freq) -> Fine Freq/1
  Phase Adjust/1(" I") -> Scaling/1(Eq-I)
  Phase Adjust/2(" Q") -> Scaling/2(Eq-Q)
  Phase Adjust/3(" SubCar Index") -> Scaling/3(Xk_index)
  Phase Adjust/4(Vout) -> Scaling/4(Vin)
  Phase Adjust/5(" Sym Index") -> Scaling/5(Sym Index)
  Convert3/1 -> Rx_debug_PhaseError/1
  Up Sample2/1 -> Rx_debug_EQ_Q/1
  Up Sample1/1 -> Rx_debug_EQ_I/1
  Up Sample/1 -> Rx_debug_PhaseCorrect/1
  Convert1/1 -> Up Sample/1
  Convert5/1 -> Up Sample2/1
  Convert4/1 -> Up Sample1/1

== system ofdm_txrx_mimo_2x/OFDM Rx MIMO/FFT & Chan Est ==
  note: Payload->WE latency was 1 - why?
  Payload : Inport
  AntA I : Inport  Port=2
  AntA Q : Inport  Port=3
  AntB I : Inport  Port=4
  AntB Q : Inport  Port=5
  Addr Gen1 : Subsystem
  Addr_Generator : Subsystem
  Assert : xbsIndex_r4/Assert  Orientation=left  assert_type=off  type_source=Explicitly  arith_type="Signed  (2's comp)"  n_bits=16  bin_pt=14  assert_rate=on  rate_source=Explicitly  period=1  output_port=on  block_type=assert
      settings: infoedit=Asserts a user-defined sample rate and/or type on a signal.<P><P>Hardware notes: In hardware this block costs nothing.  block_version=VER_STRING_GOES_HERE
  Channel Estimation : Subsystem
  Delay{1..4} (4 alike) : xbsIndex_r4/Delay  en=off  latency=1  dbl_ovrd=off  reg_retiming=off  block_type=delay
      settings: infoedit=Hardware notes: A delay line is a chain, each link of which is an SRL16 followed by a flip-flop. If register retiming is enabled, the delay line is a chain of flip-flops.  block_version=VER_STRING_GOES_HERE
  Down Sample : xbsIndex_r4/Down Sample  Orientation=left  sample_ratio=2  sample_phase=First Value of Frame  en=off  latency=0  dbl_ovrd=off  block_type=dsamp
      settings: block_version=VER_STRING_GOES_HERE
  FFT : Subsystem
  Input Buffers : Subsystem
  Output Buffers : Subsystem
  Rx Buffers Input : Scope  NumInputPorts=6
      settings: TimeRange=25000  YMin=0~-0.2~-0.2~-0.2~-0.2~0  YMax=1~0.2~0.3~0.2~0.2~1  SaveName=ScopeData46
  Symbol Counter : Subsystem
  done{1..2,7..10} (6 alike) : xbsIndex_r4/Gateway Out  hdl_port=off  timing_constraint=None  locs_specified=off  LOCs={}  block_type=gatewayout
      settings: infoedit="Gateway out block.  Converts Xilinx fixed point inputs into ouputs of type Simulink integer, double, or fixed point.<P><P>Hardware notes:  In hardware these blocks become top level output ports or are discarded, depending on how they are configured."  xl_area=[0 0 0 0 1 0 0]  block_version=VER_STRING_GOES_HERE
  Y_I : Outport
  Y_Q : Outport  Port=2
  H-I : Outport  Port=3
  H-Q : Outport  Port=4
  SubCar Index : Outport  Port=5
  Sym Index : Outport  Port=6
  Vout : Outport  Port=7
  tag scope: FFT_I=global
  wires:
  done1/1 -> Rx Buffers Input/6
  done2/1 -> Rx Buffers Input/5
  done10/1 -> Rx Buffers Input/4
  done7/1 -> Rx Buffers Input/3
  done9/1 -> Rx Buffers Input/2
  done8/1 -> Rx Buffers Input/1
  Delay1/1 -> done1/1, Input Buffers/5(We)
  FFT/5(Done) -> Symbol Counter/1(FFT Done), Addr Gen1/1(FFT Done)
  Addr_Generator/3(Ant Sel) -> Input Buffers/8(Ant Sel)
  Assert/1 -> Addr_Generator/2(FFT Xn_index)
  AntB Q/1 -> done2/1, Input Buffers/4(AntB Q)
  AntB I/1 -> done10/1, Input Buffers/3(AntB I)
  AntA Q/1 -> done7/1, Input Buffers/2(AntA Q)
  AntA I/1 -> done9/1, Input Buffers/1(AntA I)
  Payload/1 -> Symbol Counter/2(Payload), Addr Gen1/2(Payload), done8/1, Delay1/1, Addr_Generator/1(Payload)
  Addr_Generator/2(Read Addr) -> Input Buffers/7(Rd Addr)
  Addr_Generator/4(FFT Start) -> FFT/3(Start)
  Input Buffers/2(" Q") -> FFT/2(xn_Q)
  Input Buffers/1(" I") -> FFT/1(xn_I)
  Addr_Generator/1(Write Addr) -> Input Buffers/6(Wr Addr)
  Delay4/1 -> SubCar Index/1
  Delay2/1 -> Vout/1
  Output Buffers/2(" Q") -> Y_Q/1
  Channel Estimation/2(H-Q) -> H-Q/1
  Channel Estimation/1(H-I) -> H-I/1
  Output Buffers/1(" I") -> Y_I/1
  Channel Estimation/3(Est Vout) -> Down Sample/1
  Symbol Counter/1(Sym Index) -> Channel Estimation/5(Sym Index), Output Buffers/5(SymIndex)
  FFT/4(Vout) -> Channel Estimation/4(Vin), Output Buffers/4(FFT Vout)
  FFT/3(xk_Index) -> Channel Estimation/3(Xk Index), Output Buffers/3(Wr Addr)
  FFT/2(xk_Q) -> Channel Estimation/2(Q), Output Buffers/2(Q)
  FFT/1(xk_I) -> Channel Estimation/1(I), Output Buffers/1(I), [FFT_I]
  FFT/6(xn_Index) -> Assert/1
  Down Sample/1 -> Addr Gen1/3(NotTraining)
  Addr Gen1/2(Rd Addr) -> Output Buffers/6(Rd Addr), Channel Estimation/6(Est Index), Delay4/1
  Addr Gen1/3(Vout) -> Delay2/1
  Delay3/1 -> Sym Index/1
  Addr Gen1/1(SymIndex_out) -> Delay3/1

== system ofdm_txrx_mimo_2x/OFDM Rx MIMO/Long Corr 4x ==
  note: changed latency to zero
  note: changed latency to 0
  I In : Inport
  Q In : Inport  Port=2
  AddSub : xbsIndex_r4/AddSub  mode=Subtraction  use_carryin=off  use_carryout=off  en=off  latency=0  precision=Full  arith_type=Unsigned  n_bits=8  bin_pt=2  quantization=Truncate  overflow=Wrap  dbl_ovrd=off  use_behavioral_HDL=on  pipelined=off  use_rpm=off  hw_selection=Fabric  block_type=addsub
      settings: xl_area=[5 0 0 8 0 0 0]  block_version=VER_STRING_GOES_HERE
  AddSub1 : xbsIndex_r4/AddSub  mode=Addition  use_carryin=off  use_carryout=off  en=off  latency=0  precision=Full  arith_type=Unsigned  n_bits=8  bin_pt=2  quantization=Truncate  overflow=Wrap  dbl_ovrd=off  use_behavioral_HDL=on  pipelined=off  use_rpm=off  hw_selection=Fabric  block_type=addsub
      settings: xl_area=[5 0 0 8 0 0 0]  block_version=VER_STRING_GOES_HERE
  AddSub2 : xbsIndex_r4/AddSub  mode=Addition  use_carryin=off  use_carryout=off  en=off  latency=0  precision=Full  arith_type=Unsigned  n_bits=8  bin_pt=2  quantization=Truncate  overflow=Wrap  dbl_ovrd=off  use_behavioral_HDL=on  pipelined=off  use_rpm=off  hw_selection=Fabric  block_type=addsub
      settings: xl_area=[10 0 0 18 0 0 0]  block_version=VER_STRING_GOES_HERE
  C1 : Subsystem  AncestorBlock=ofdm_rx_pom36_cfglib/correlator2/Original Subsystem/C1  mask{ns=0, N=4, h=hr(1:65), Ts_In=4}
      mask prompts: ns "Num sub-correlator"; N "Coefficients/correlator"; h "Coefficients"; Ts_In "Input sample period"
  C4 : Subsystem  AncestorBlock=ofdm_rx_pom36_cfglib/correlator2/Original Subsystem/C1  mask{ns=0, N=4, h=hi(1:65), Ts_In=4}
      mask prompts: ns "Num sub-correlator"; N "Coefficients/correlator"; h "Coefficients"; Ts_In "Input sample period"
  C6 : Subsystem  AncestorBlock=ofdm_rx_pom36_cfglib/correlator2/Original Subsystem/C1  mask{ns=0, N=4, h=hi(1:65), Ts_In=4}
      mask prompts: ns "Num sub-correlator"; N "Coefficients/correlator"; h "Coefficients"; Ts_In "Input sample period"
  C7 : Subsystem  AncestorBlock=ofdm_rx_pom36_cfglib/correlator2/Original Subsystem/C1  mask{ns=0, N=4, h=hr(1:65), Ts_In=4}
      mask prompts: ns "Num sub-correlator"; N "Coefficients/correlator"; h "Coefficients"; Ts_In "Input sample period"
  Gateway Out{3..8} (6 alike) : xbsIndex_r4/Gateway Out  hdl_port=off  timing_constraint=None  locs_specified=off  LOCs={}  block_type=gatewayout
      settings: infoedit="Gateway out block.  Converts Xilinx fixed point inputs into ouputs of type Simulink integer, double, or fixed point.<P><P>Hardware notes:  In hardware these blocks become top level output ports or are discarded, depending on how they are configured."  xl_area=[0 0 0 0 0 0 0]  block_version=VER_STRING_GOES_HERE
  Long Corr Scope4x : Scope  NumInputPorts=6
      settings: YMin=-1~-1~-30~-30~-20~-25  YMax=1~1~70~70~20~20  SaveName=ScopeData2
  Mult : xbsIndex_r4/Mult  precision=Full  arith_type="Signed  (2's comp)"  n_bits=8  bin_pt=2  quantization=Truncate  overflow=Wrap  en=off  latency=1  dbl_ovrd=off  use_behavioral_HDL=off  use_embedded=on  opt=Speed  optimum_pipeline=off  pipeline=on  use_rpm=off  placement_style=Rectangular shape  block_type=mult
      settings: infoedit=Hardware notes: To use the internal pipeline stage of the dedicated multiplier you must select 'Pipeline for maximum performance'.  xl_area=[60 18 0 106 0 0 0]  block_version=VER_STRING_GOES_HERE
  Mult1 : xbsIndex_r4/Mult  precision=Full  arith_type="Signed  (2's comp)"  n_bits=8  bin_pt=2  quantization=Truncate  overflow=Wrap  en=off  latency=1  dbl_ovrd=off  use_behavioral_HDL=off  use_embedded=on  opt=Speed  optimum_pipeline=off  pipeline=on  use_rpm=off  placement_style=Rectangular shape  block_type=mult
      settings: infoedit=Hardware notes: To use the internal pipeline stage of the dedicated multiplier you must select 'Pipeline for maximum performance'.  xl_area=[60 18 0 106 0 0 0]  block_version=VER_STRING_GOES_HERE
  Threshold1 : xbsIndex_r4/Threshold  en=off  latency=0  dbl_ovrd=off  block_type=sgn
      settings: infoedit="Presents -1 as output if input is negative; otherwise presents 1.  Output is a two bit signed number."  xl_area=[0 0 0 0 0 0 0]  block_version=VER_STRING_GOES_HERE
  Threshold2 : xbsIndex_r4/Threshold  en=off  latency=0  dbl_ovrd=off  block_type=sgn
      settings: infoedit="Presents -1 as output if input is negative; otherwise presents 1.  Output is a two bit signed number."  xl_area=[0 0 0 0 0 0 0]  block_version=VER_STRING_GOES_HERE
  y : Outport
  wires:
  Gateway Out8/1 -> Long Corr Scope4x/5
  Gateway Out7/1 -> Long Corr Scope4x/4
  Gateway Out6/1 -> Long Corr Scope4x/3
  Gateway Out5/1 -> Long Corr Scope4x/6
  Gateway Out4/1 -> Long Corr Scope4x/2
  Gateway Out3/1 -> Long Corr Scope4x/1
  Threshold1/1 -> C4/1(x), C7/1(x)
  Threshold2/1 -> C1/1(x), C6/1(x)
  I In/1 -> Gateway Out3/1, Threshold2/1
  Q In/1 -> Gateway Out4/1, Threshold1/1
  C4/1(y) -> Gateway Out7/1, AddSub/2
  C6/1(y) -> Gateway Out8/1, AddSub1/1
  C7/1(y) -> Gateway Out5/1, AddSub1/2
  AddSub/1 -> Mult/1, Mult/2
  AddSub1/1 -> Mult1/1, Mult1/2
  Mult/1 -> AddSub2/1
  Mult1/1 -> AddSub2/2
  AddSub2/1 -> y/1
  C1/1(y) -> Gateway Out6/1, AddSub/1
